simulink model "VirtualADAPT"  (Simulink 8.9)
settings: Solver=ode45  StartTime=0.0  StopTime=inf  RelTol=1e-3  EnableMultiTasking=on
PostLoadFcn:
  | FaultInjectionGUIHandle = FaultInjectionGUI;
InitFcn:
  | VirtualADAPTDataStructure
undefined symbols (not defined in the file or in its folder): ln
libraries: simulink x363
(part 12 of 26)

== system VirtualADAPT/VirtualADAPTv1/PowerDistribution/Inverter2/VoutSetpoint/Fault Inject ==
  Nominal : Inport
  Fault Profile : Inport  Port=2
  Step : Inport  Port=3
  Ramp : Inport  Port=4
  Bias : Inport  Port=5
  stuckAt : Inport  Port=6
  Switch1 : MultiPortSwitch  Inputs=5  SaturateOnIntegerOverflow=on  InputSameDT=on
  Out1 : Outport
  wires:
  Nominal/1 -> Switch1/2
  Fault Profile/1 -> Switch1/1
  Step/1 -> Switch1/3
  Ramp/1 -> Switch1/4
  Bias/1 -> Switch1/5
  stuckAt/1 -> Switch1/6
  Switch1/1 -> Out1/1

== system VirtualADAPT/VirtualADAPTv1/PowerDistribution/Inverter2/Z1/determineState ==
  In1 : Inport
  In2 : Inport  Port=2
  Fcn : Fcn  Expr=(!u(1)&&u(2)) || (u(1)&&(!u(3)))
  Memory : Memory  X0=1
  Mux : Mux  Inputs=3
  Out1 : Outport
  wires:
  Memory/1 -> Mux/1
  In1/1 -> Mux/2
  In2/1 -> Mux/3
  Mux/1 -> Fcn/1
  Fcn/1 -> Memory/1, Out1/1

== system VirtualADAPT/VirtualADAPTv1/PowerDistribution/Inverter2/Z1Delay/C ==
  F : Inport
  Capacitance : Constant  Value=C
  Fault Inject : Subsystem
  Fault Profile : Constant  Value=FP
  InitialCondition : Constant  Value=IC
  Integrator : Integrator  InitialConditionSource=external
  Product1 : Product  SaturateOnIntegerOverflow=on  RndMeth=Zero  InputSameDT=on  OutDataTypeStr=Inherit: Same as first input
  Product2 : Product  SaturateOnIntegerOverflow=on  RndMeth=Zero  InputSameDT=on  OutDataTypeStr=Inherit: Same as first input
  Ramp : simulink/Sources/Ramp  slope=FM  start=FT  X0=C
  Reciprocal : Math  Operator=reciprocal  SaturateOnIntegerOverflow=on
  Step : Step  Time=FT  Before=C  After=(1 + FM)*C  SampleTime=0
  E : Outport
  C : Outport  Port=2
  q : Outport  Port=3
  wires:
  F/1 -> Integrator/1
  InitialCondition/1 -> Product1/1
  Capacitance/1 -> Fault Inject/1(Nominal), Product1/2
  Product1/1 -> Integrator/2
  Integrator/1 -> Product2/1, q/1
  Fault Inject/1(Out1) -> Reciprocal/1, C/1
  Reciprocal/1 -> Product2/2
  Fault Profile/1 -> Fault Inject/2(Fault Profile)
  Step/1 -> Fault Inject/3(Step)
  Ramp/1 -> Fault Inject/4(Ramp)
  Product2/1 -> E/1

== system VirtualADAPT/VirtualADAPTv1/PowerDistribution/Inverter2/Z1Delay/OneJunction ==
  u(1) : Inport
  u(2) : Inport  Port=2
  u(3) : Inport  Port=3
  Adder : Sum  Inputs=-+  SaturateOnIntegerOverflow=on  InputSameDT=on  OutDataTypeStr=Inherit: Same as first input
  Gain : Gain  SaturateOnIntegerOverflow=on  OutDataTypeStr=Inherit: Same as input  ParamDataTypeStr=Inherit: Same as input
  y(1) : Outport
  y(2) : Outport  Port=2
  y(3) : Outport  Port=3
  O : Outport  Port=4
  wires:
  Adder/1 -> Gain/1
  u(2)/1 -> y(1)/1, y(3)/1, O/1
  u(1)/1 -> Adder/1
  Gain/1 -> y(2)/1
  u(3)/1 -> Adder/2

== system VirtualADAPT/VirtualADAPTv1/PowerDistribution/Inverter2/Z1Delay/R ==
  In : Inport
  Bond : Constant  Value=2
  Computing R : Subsystem
  Fault Profile : Constant  Value=FP
  JuncDB : Constant  Value=2
  Junction Type : Constant
  Ramp : simulink/Sources/Ramp  slope=FM  start=FT  X0=R
  Resistor Value : Constant  Value=R
  Step : Step  Time=FT  Before=R  After=(1 + FM)*R  SampleTime=0
  Out : Outport
  R : Outport  Port=2
  wires:
  Computing R/1(Out1) -> Out/1
  In/1 -> Computing R/1(In1)
  Resistor Value/1 -> Computing R/2(RNominal)
  Fault Profile/1 -> Computing R/3(Fault Profile)
  Step/1 -> Computing R/4(Step)
  Ramp/1 -> Computing R/5(Ramp)
  JuncDB/1 -> Computing R/6(JuncDB)
  Bond/1 -> Computing R/7(Bond)
  Junction Type/1 -> Computing R/8(Junction Type)
  Computing R/2(Out2) -> R/1

== system VirtualADAPT/VirtualADAPTv1/PowerDistribution/Inverter2/Z1Delay/Se ==
  F : Inport
  Se : Inport  Port=2
  Bias : Step  Time=FT  After=FM  SampleTime=0
  Fault Inject : Subsystem
  Fault Profile : Constant  Value=FP
  Mult2 : Product  SaturateOnIntegerOverflow=on  RndMeth=Zero  InputSameDT=on  OutDataTypeStr=Inherit: Same as first input
  Multiply : Product  SaturateOnIntegerOverflow=on  RndMeth=Zero  InputSameDT=on  OutDataTypeStr=Inherit: Same as first input
  Ramp : simulink/Sources/Ramp  slope=FM  start=FT  X0=0
  Step : Step  Time=FT  Before=1  After=(1 + FM)  SampleTime=0
  StuckAt : Step  Time=FT  Before=1  After=0  SampleTime=0
  StuckAt2 : Step  Time=FT  After=FM  SampleTime=0
  Sum : Sum  SaturateOnIntegerOverflow=on  InputSameDT=on  OutDataTypeStr=Inherit: Same as first input
  Sum2 : Sum  SaturateOnIntegerOverflow=on  InputSameDT=on  OutDataTypeStr=Inherit: Same as first input
  Sum3 : Sum  SaturateOnIntegerOverflow=on  InputSameDT=on  OutDataTypeStr=Inherit: Same as first input
  E : Outport
  wires:
  Fault Inject/1(Out1) -> E/1
  Se/1 -> Fault Inject/1(Nominal), Multiply/1, Sum/1, Sum2/1, Mult2/1
  Fault Profile/1 -> Fault Inject/2(Fault Profile)
  Step/1 -> Multiply/2
  Ramp/1 -> Sum/2
  Multiply/1 -> Fault Inject/3(Step)
  Sum/1 -> Fault Inject/4(Ramp)
  Bias/1 -> Sum2/2
  Sum2/1 -> Fault Inject/5(Bias)
  StuckAt/1 -> Mult2/2
  Mult2/1 -> Sum3/1
  StuckAt2/1 -> Sum3/2
  Sum3/1 -> Fault Inject/6(stuckAt)

== system VirtualADAPT/VirtualADAPTv1/PowerDistribution/Inverter2/Z1Delay/SignalModFunc ==
  u(1) : Inport
  Function : Fcn  Expr=u(1)
  Mux : Mux  Inputs=1
  y(1) : Outport
  wires:
  u(1)/1 -> Mux/1
  Mux/1 -> Function/1
  Function/1 -> y(1)/1

== system VirtualADAPT/VirtualADAPTv1/PowerDistribution/Inverter2/Z1Delay/ZeroJunction ==
  u(1) : Inport
  u(2) : Inport  Port=2
  Adder : Sum  Inputs=+  SaturateOnIntegerOverflow=on  InputSameDT=on  OutDataTypeStr=Inherit: Same as first input
  Gain : Gain  SaturateOnIntegerOverflow=on  OutDataTypeStr=Inherit: Same as input  ParamDataTypeStr=Inherit: Same as input
  y(1) : Outport
  y(2) : Outport  Port=2
  O : Outport  Port=3
  wires:
  Adder/1 -> Gain/1
  Gain/1 -> y(1)/1
  u(1)/1 -> y(2)/1, O/1
  u(2)/1 -> Adder/1

== system VirtualADAPT/VirtualADAPTv1/PowerDistribution/Inverter2/ZDel/determineState ==
  In1 : Inport
  In2 : Inport  Port=2
  Fcn : Fcn  Expr=(!u(1)&&u(2)) || (u(1)&&(!u(3)))
  Memory : Memory
  Mux : Mux  Inputs=3
  Out1 : Outport
  wires:
  Memory/1 -> Mux/1
  In1/1 -> Mux/2
  In2/1 -> Mux/3
  Mux/1 -> Fcn/1
  Fcn/1 -> Memory/1, Out1/1

== system VirtualADAPT/VirtualADAPTv1/PowerDistribution/Inverter2/ZForR1/determineState ==
  In1 : Inport
  In2 : Inport  Port=2
  Fcn : Fcn  Expr=(!u(1)&&u(2)) || (u(1)&&(!u(3)))
  Memory : Memory  X0=1
  Mux : Mux  Inputs=3
  Out1 : Outport
  wires:
  Memory/1 -> Mux/1
  In1/1 -> Mux/2
  In2/1 -> Mux/3
  Mux/1 -> Fcn/1
  Fcn/1 -> Memory/1, Out1/1

== system VirtualADAPT/VirtualADAPTv1/PowerDistribution/Inverter2/ZForR2/determineState ==
  In1 : Inport
  In2 : Inport  Port=2
  Fcn : Fcn  Expr=(!u(1)&&u(2)) || (u(1)&&(!u(3)))
  Memory : Memory
  Mux : Mux  Inputs=3
  Out1 : Outport
  wires:
  Memory/1 -> Mux/1
  In1/1 -> Mux/2
  In2/1 -> Mux/3
  Mux/1 -> Fcn/1
  Fcn/1 -> Memory/1, Out1/1

== system VirtualADAPT/VirtualADAPTv1/PowerDistribution/Inverter2/ZFreq/determineState ==
  In1 : Inport
  In2 : Inport  Port=2
  Fcn : Fcn  Expr=(!u(1)&&u(2)) || (u(1)&&(!u(3)))
  Memory : Memory
  Mux : Mux  Inputs=3
  Out1 : Outport
  wires:
  Memory/1 -> Mux/1
  In1/1 -> Mux/2
  In2/1 -> Mux/3
  Mux/1 -> Fcn/1
  Fcn/1 -> Memory/1, Out1/1

== system VirtualADAPT/VirtualADAPTv1/PowerDistribution/Inverter2/ZVout/determineState ==
  In1 : Inport
  In2 : Inport  Port=2
  Fcn : Fcn  Expr=(!u(1)&&u(2)) || (u(1)&&(!u(3)))
  Memory : Memory
  Mux : Mux  Inputs=3
  Out1 : Outport
  wires:
  Memory/1 -> Mux/1
  In1/1 -> Mux/2
  In2/1 -> Mux/3
  Mux/1 -> Fcn/1
  Fcn/1 -> Memory/1, Out1/1

== system VirtualADAPT/VirtualADAPTv1/PowerDistribution/SensorE165/MSe/Fault Inject ==
  Nominal : Inport
  Fault Profile : Inport  Port=2
  Step : Inport  Port=3
  Ramp : Inport  Port=4
  Bias : Inport  Port=5
  stuckAt : Inport  Port=6
  Switch1 : MultiPortSwitch  Inputs=5  SaturateOnIntegerOverflow=on  InputSameDT=on
  Out1 : Outport
  wires:
  Nominal/1 -> Switch1/2
  Fault Profile/1 -> Switch1/1
  Step/1 -> Switch1/3
  Ramp/1 -> Switch1/4
  Bias/1 -> Switch1/5
  stuckAt/1 -> Switch1/6
  Switch1/1 -> Out1/1

== system VirtualADAPT/VirtualADAPTv1/PowerDistribution/SensorE165/R/Computing R ==
  In1 : Inport
  RNominal : Inport  Port=2
  Fault Profile : Inport  Port=3
  Step : Inport  Port=4
  Ramp : Inport  Port=5
  JuncDB : Inport  Port=6
  Bond : Inport  Port=7
  Junction Type : Inport  Port=8
  CalcCausality : Fcn  Expr=(u(1) == u(2) && u(3) ==1) || (u(1) !=u(2) && u(3) == 0)
  CalcOutput : Fcn  Expr=(u(1)*u(2)^u(3))
  MinusOne : Constant  Value=-1
  Mux1 : Mux  Inputs=3
  Mux2 : Mux  Inputs=3
  One : Constant
  Switch1 : MultiPortSwitch  SaturateOnIntegerOverflow=on  InputSameDT=on
  Switch2 : MultiPortSwitch  DataPortOrder=Zero-based contiguous  Inputs=2  zeroidx=on  SaturateOnIntegerOverflow=on  InputSameDT=on
  Out1 : Outport
  Out2 : Outport  Port=2
  wires:
  In1/1 -> Mux2/1
  Fault Profile/1 -> Switch1/1
  RNominal/1 -> Switch1/2
  Step/1 -> Switch1/3
  Ramp/1 -> Switch1/4
  Switch1/1 -> Out2/1, Mux2/2
  JuncDB/1 -> Mux1/1
  Bond/1 -> Mux1/2
  Junction Type/1 -> Mux1/3
  Mux1/1 -> CalcCausality/1
  CalcCausality/1 -> Switch2/1
  One/1 -> Switch2/2
  MinusOne/1 -> Switch2/3
  Switch2/1 -> Mux2/3
  Mux2/1 -> CalcOutput/1
  CalcOutput/1 -> Out1/1

== system VirtualADAPT/VirtualADAPTv1/PowerDistribution/SensorE167/MSe/Fault Inject ==
  Nominal : Inport
  Fault Profile : Inport  Port=2
  Step : Inport  Port=3
  Ramp : Inport  Port=4
  Bias : Inport  Port=5
  stuckAt : Inport  Port=6
  Switch1 : MultiPortSwitch  Inputs=5  SaturateOnIntegerOverflow=on  InputSameDT=on
  Out1 : Outport
  wires:
  Nominal/1 -> Switch1/2
  Fault Profile/1 -> Switch1/1
  Step/1 -> Switch1/3
  Ramp/1 -> Switch1/4
  Bias/1 -> Switch1/5
  stuckAt/1 -> Switch1/6
  Switch1/1 -> Out1/1

== system VirtualADAPT/VirtualADAPTv1/PowerDistribution/SensorE167/R/Computing R ==
  In1 : Inport
  RNominal : Inport  Port=2
  Fault Profile : Inport  Port=3
  Step : Inport  Port=4
  Ramp : Inport  Port=5
  JuncDB : Inport  Port=6
  Bond : Inport  Port=7
  Junction Type : Inport  Port=8
  CalcCausality : Fcn  Expr=(u(1) == u(2) && u(3) ==1) || (u(1) !=u(2) && u(3) == 0)
  CalcOutput : Fcn  Expr=(u(1)*u(2)^u(3))
  MinusOne : Constant  Value=-1
  Mux1 : Mux  Inputs=3
  Mux2 : Mux  Inputs=3
  One : Constant
  Switch1 : MultiPortSwitch  SaturateOnIntegerOverflow=on  InputSameDT=on
  Switch2 : MultiPortSwitch  DataPortOrder=Zero-based contiguous  Inputs=2  zeroidx=on  SaturateOnIntegerOverflow=on  InputSameDT=on
  Out1 : Outport
  Out2 : Outport  Port=2
  wires:
  In1/1 -> Mux2/1
  Fault Profile/1 -> Switch1/1
  RNominal/1 -> Switch1/2
  Step/1 -> Switch1/3
  Ramp/1 -> Switch1/4
  Switch1/1 -> Out2/1, Mux2/2
  JuncDB/1 -> Mux1/1
  Bond/1 -> Mux1/2
  Junction Type/1 -> Mux1/3
  Mux1/1 -> CalcCausality/1
  CalcCausality/1 -> Switch2/1
  One/1 -> Switch2/2
  MinusOne/1 -> Switch2/3
  Switch2/1 -> Mux2/3
  Mux2/1 -> CalcOutput/1
  CalcOutput/1 -> Out1/1

== system VirtualADAPT/VirtualADAPTv1/PowerDistribution/SensorE181/MSe/Fault Inject ==
  Nominal : Inport
  Fault Profile : Inport  Port=2
  Step : Inport  Port=3
  Ramp : Inport  Port=4
  Bias : Inport  Port=5
  stuckAt : Inport  Port=6
  Switch1 : MultiPortSwitch  Inputs=5  SaturateOnIntegerOverflow=on  InputSameDT=on
  Out1 : Outport
  wires:
  Nominal/1 -> Switch1/2
  Fault Profile/1 -> Switch1/1
  Step/1 -> Switch1/3
  Ramp/1 -> Switch1/4
  Bias/1 -> Switch1/5
  stuckAt/1 -> Switch1/6
  Switch1/1 -> Out1/1

== system VirtualADAPT/VirtualADAPTv1/PowerDistribution/SensorE181/R/Computing R ==
  In1 : Inport
  RNominal : Inport  Port=2
  Fault Profile : Inport  Port=3
  Step : Inport  Port=4
  Ramp : Inport  Port=5
  JuncDB : Inport  Port=6
  Bond : Inport  Port=7
  Junction Type : Inport  Port=8
  CalcCausality : Fcn  Expr=(u(1) == u(2) && u(3) ==1) || (u(1) !=u(2) && u(3) == 0)
  CalcOutput : Fcn  Expr=(u(1)*u(2)^u(3))
  MinusOne : Constant  Value=-1
  Mux1 : Mux  Inputs=3
  Mux2 : Mux  Inputs=3
  One : Constant
  Switch1 : MultiPortSwitch  SaturateOnIntegerOverflow=on  InputSameDT=on
  Switch2 : MultiPortSwitch  DataPortOrder=Zero-based contiguous  Inputs=2  zeroidx=on  SaturateOnIntegerOverflow=on  InputSameDT=on
  Out1 : Outport
  Out2 : Outport  Port=2
  wires:
  In1/1 -> Mux2/1
  Fault Profile/1 -> Switch1/1
  RNominal/1 -> Switch1/2
  Step/1 -> Switch1/3
  Ramp/1 -> Switch1/4
  Switch1/1 -> Out2/1, Mux2/2
  JuncDB/1 -> Mux1/1
  Bond/1 -> Mux1/2
  Junction Type/1 -> Mux1/3
  Mux1/1 -> CalcCausality/1
  CalcCausality/1 -> Switch2/1
  One/1 -> Switch2/2
  MinusOne/1 -> Switch2/3
  Switch2/1 -> Mux2/3
  Mux2/1 -> CalcOutput/1
  CalcOutput/1 -> Out1/1

== system VirtualADAPT/VirtualADAPTv1/PowerDistribution/SensorE265/MSe/Fault Inject ==
  Nominal : Inport
  Fault Profile : Inport  Port=2
  Step : Inport  Port=3
  Ramp : Inport  Port=4
  Bias : Inport  Port=5
  stuckAt : Inport  Port=6
  Switch1 : MultiPortSwitch  Inputs=5  SaturateOnIntegerOverflow=on  InputSameDT=on
  Out1 : Outport
  wires:
  Nominal/1 -> Switch1/2
  Fault Profile/1 -> Switch1/1
  Step/1 -> Switch1/3
  Ramp/1 -> Switch1/4
  Bias/1 -> Switch1/5
  stuckAt/1 -> Switch1/6
  Switch1/1 -> Out1/1

== system VirtualADAPT/VirtualADAPTv1/PowerDistribution/SensorE265/R/Computing R ==
  In1 : Inport
  RNominal : Inport  Port=2
  Fault Profile : Inport  Port=3
  Step : Inport  Port=4
  Ramp : Inport  Port=5
  JuncDB : Inport  Port=6
  Bond : Inport  Port=7
  Junction Type : Inport  Port=8
  CalcCausality : Fcn  Expr=(u(1) == u(2) && u(3) ==1) || (u(1) !=u(2) && u(3) == 0)
  CalcOutput : Fcn  Expr=(u(1)*u(2)^u(3))
  MinusOne : Constant  Value=-1
  Mux1 : Mux  Inputs=3
  Mux2 : Mux  Inputs=3
  One : Constant
  Switch1 : MultiPortSwitch  SaturateOnIntegerOverflow=on  InputSameDT=on
  Switch2 : MultiPortSwitch  DataPortOrder=Zero-based contiguous  Inputs=2  zeroidx=on  SaturateOnIntegerOverflow=on  InputSameDT=on
  Out1 : Outport
  Out2 : Outport  Port=2
  wires:
  In1/1 -> Mux2/1
  Fault Profile/1 -> Switch1/1
  RNominal/1 -> Switch1/2
  Step/1 -> Switch1/3
  Ramp/1 -> Switch1/4
  Switch1/1 -> Out2/1, Mux2/2
  JuncDB/1 -> Mux1/1
  Bond/1 -> Mux1/2
  Junction Type/1 -> Mux1/3
  Mux1/1 -> CalcCausality/1
  CalcCausality/1 -> Switch2/1
  One/1 -> Switch2/2
  MinusOne/1 -> Switch2/3
  Switch2/1 -> Mux2/3
  Mux2/1 -> CalcOutput/1
  CalcOutput/1 -> Out1/1

== system VirtualADAPT/VirtualADAPTv1/PowerDistribution/SensorE267/MSe/Fault Inject ==
  Nominal : Inport
  Fault Profile : Inport  Port=2
  Step : Inport  Port=3
  Ramp : Inport  Port=4
  Bias : Inport  Port=5
  stuckAt : Inport  Port=6
  Switch1 : MultiPortSwitch  Inputs=5  SaturateOnIntegerOverflow=on  InputSameDT=on
  Out1 : Outport
  wires:
  Nominal/1 -> Switch1/2
  Fault Profile/1 -> Switch1/1
  Step/1 -> Switch1/3
  Ramp/1 -> Switch1/4
  Bias/1 -> Switch1/5
  stuckAt/1 -> Switch1/6
  Switch1/1 -> Out1/1

== system VirtualADAPT/VirtualADAPTv1/PowerDistribution/SensorE267/R/Computing R ==
  In1 : Inport
  RNominal : Inport  Port=2
  Fault Profile : Inport  Port=3
  Step : Inport  Port=4
  Ramp : Inport  Port=5
  JuncDB : Inport  Port=6
  Bond : Inport  Port=7
  Junction Type : Inport  Port=8
  CalcCausality : Fcn  Expr=(u(1) == u(2) && u(3) ==1) || (u(1) !=u(2) && u(3) == 0)
  CalcOutput : Fcn  Expr=(u(1)*u(2)^u(3))
  MinusOne : Constant  Value=-1
  Mux1 : Mux  Inputs=3
  Mux2 : Mux  Inputs=3
  One : Constant
  Switch1 : MultiPortSwitch  SaturateOnIntegerOverflow=on  InputSameDT=on
  Switch2 : MultiPortSwitch  DataPortOrder=Zero-based contiguous  Inputs=2  zeroidx=on  SaturateOnIntegerOverflow=on  InputSameDT=on
  Out1 : Outport
  Out2 : Outport  Port=2
  wires:
  In1/1 -> Mux2/1
  Fault Profile/1 -> Switch1/1
  RNominal/1 -> Switch1/2
  Step/1 -> Switch1/3
  Ramp/1 -> Switch1/4
  Switch1/1 -> Out2/1, Mux2/2
  JuncDB/1 -> Mux1/1
  Bond/1 -> Mux1/2
  Junction Type/1 -> Mux1/3
  Mux1/1 -> CalcCausality/1
  CalcCausality/1 -> Switch2/1
  One/1 -> Switch2/2
  MinusOne/1 -> Switch2/3
  Switch2/1 -> Mux2/3
  Mux2/1 -> CalcOutput/1
  CalcOutput/1 -> Out1/1

== system VirtualADAPT/VirtualADAPTv1/PowerDistribution/SensorE281/MSe/Fault Inject ==
  Nominal : Inport
  Fault Profile : Inport  Port=2
  Step : Inport  Port=3
  Ramp : Inport  Port=4
  Bias : Inport  Port=5
  stuckAt : Inport  Port=6
  Switch1 : MultiPortSwitch  Inputs=5  SaturateOnIntegerOverflow=on  InputSameDT=on
  Out1 : Outport
  wires:
  Nominal/1 -> Switch1/2
  Fault Profile/1 -> Switch1/1
  Step/1 -> Switch1/3
  Ramp/1 -> Switch1/4
  Bias/1 -> Switch1/5
  stuckAt/1 -> Switch1/6
  Switch1/1 -> Out1/1

== system VirtualADAPT/VirtualADAPTv1/PowerDistribution/SensorE281/R/Computing R ==
  In1 : Inport
  RNominal : Inport  Port=2
  Fault Profile : Inport  Port=3
  Step : Inport  Port=4
  Ramp : Inport  Port=5
  JuncDB : Inport  Port=6
  Bond : Inport  Port=7
  Junction Type : Inport  Port=8
  CalcCausality : Fcn  Expr=(u(1) == u(2) && u(3) ==1) || (u(1) !=u(2) && u(3) == 0)
  CalcOutput : Fcn  Expr=(u(1)*u(2)^u(3))
  MinusOne : Constant  Value=-1
  Mux1 : Mux  Inputs=3
  Mux2 : Mux  Inputs=3
  One : Constant
  Switch1 : MultiPortSwitch  SaturateOnIntegerOverflow=on  InputSameDT=on
  Switch2 : MultiPortSwitch  DataPortOrder=Zero-based contiguous  Inputs=2  zeroidx=on  SaturateOnIntegerOverflow=on  InputSameDT=on
  Out1 : Outport
  Out2 : Outport  Port=2
  wires:
  In1/1 -> Mux2/1
  Fault Profile/1 -> Switch1/1
  RNominal/1 -> Switch1/2
  Step/1 -> Switch1/3
  Ramp/1 -> Switch1/4
  Switch1/1 -> Out2/1, Mux2/2
  JuncDB/1 -> Mux1/1
  Bond/1 -> Mux1/2
  Junction Type/1 -> Mux1/3
  Mux1/1 -> CalcCausality/1
  CalcCausality/1 -> Switch2/1
  One/1 -> Switch2/2
  MinusOne/1 -> Switch2/3
  Switch2/1 -> Mux2/3
  Mux2/1 -> CalcOutput/1
  CalcOutput/1 -> Out1/1

== system VirtualADAPT/VirtualADAPTv1/PowerDistribution/SensorISH162/MSe/Fault Inject ==
  Nominal : Inport
  Fault Profile : Inport  Port=2
  Step : Inport  Port=3
  Ramp : Inport  Port=4
  Bias : Inport  Port=5
  stuckAt : Inport  Port=6
  Switch1 : MultiPortSwitch  Inputs=5  SaturateOnIntegerOverflow=on  InputSameDT=on
  Out1 : Outport
  wires:
  Nominal/1 -> Switch1/2
  Fault Profile/1 -> Switch1/1
  Step/1 -> Switch1/3
  Ramp/1 -> Switch1/4
  Bias/1 -> Switch1/5
  stuckAt/1 -> Switch1/6
  Switch1/1 -> Out1/1

== system VirtualADAPT/VirtualADAPTv1/PowerDistribution/SensorISH162/R/Computing R ==
  In1 : Inport
  RNominal : Inport  Port=2
  Fault Profile : Inport  Port=3
  Step : Inport  Port=4
  Ramp : Inport  Port=5
  JuncDB : Inport  Port=6
  Bond : Inport  Port=7
  Junction Type : Inport  Port=8
  CalcCausality : Fcn  Expr=(u(1) == u(2) && u(3) ==1) || (u(1) !=u(2) && u(3) == 0)
  CalcOutput : Fcn  Expr=(u(1)*u(2)^u(3))
  MinusOne : Constant  Value=-1
  Mux1 : Mux  Inputs=3
  Mux2 : Mux  Inputs=3
  One : Constant
  Switch1 : MultiPortSwitch  SaturateOnIntegerOverflow=on  InputSameDT=on
  Switch2 : MultiPortSwitch  DataPortOrder=Zero-based contiguous  Inputs=2  zeroidx=on  SaturateOnIntegerOverflow=on  InputSameDT=on
  Out1 : Outport
  Out2 : Outport  Port=2
  wires:
  In1/1 -> Mux2/1
  Fault Profile/1 -> Switch1/1
  RNominal/1 -> Switch1/2
  Step/1 -> Switch1/3
  Ramp/1 -> Switch1/4
  Switch1/1 -> Out2/1, Mux2/2
  JuncDB/1 -> Mux1/1
  Bond/1 -> Mux1/2
  Junction Type/1 -> Mux1/3
  Mux1/1 -> CalcCausality/1
  CalcCausality/1 -> Switch2/1
  One/1 -> Switch2/2
  MinusOne/1 -> Switch2/3
  Switch2/1 -> Mux2/3
  Mux2/1 -> CalcOutput/1
  CalcOutput/1 -> Out1/1

== system VirtualADAPT/VirtualADAPTv1/PowerDistribution/SensorISH166/MSe/Fault Inject ==
  Nominal : Inport
  Fault Profile : Inport  Port=2
  Step : Inport  Port=3
  Ramp : Inport  Port=4
  Bias : Inport  Port=5
  stuckAt : Inport  Port=6
  Switch1 : MultiPortSwitch  Inputs=5  SaturateOnIntegerOverflow=on  InputSameDT=on
  Out1 : Outport
  wires:
  Nominal/1 -> Switch1/2
  Fault Profile/1 -> Switch1/1
  Step/1 -> Switch1/3
  Ramp/1 -> Switch1/4
  Bias/1 -> Switch1/5
  stuckAt/1 -> Switch1/6
  Switch1/1 -> Out1/1

== system VirtualADAPT/VirtualADAPTv1/PowerDistribution/SensorISH166/R/Computing R ==
  In1 : Inport
  RNominal : Inport  Port=2
  Fault Profile : Inport  Port=3
  Step : Inport  Port=4
  Ramp : Inport  Port=5
  JuncDB : Inport  Port=6
  Bond : Inport  Port=7
  Junction Type : Inport  Port=8
  CalcCausality : Fcn  Expr=(u(1) == u(2) && u(3) ==1) || (u(1) !=u(2) && u(3) == 0)
  CalcOutput : Fcn  Expr=(u(1)*u(2)^u(3))
  MinusOne : Constant  Value=-1
  Mux1 : Mux  Inputs=3
  Mux2 : Mux  Inputs=3
  One : Constant
  Switch1 : MultiPortSwitch  SaturateOnIntegerOverflow=on  InputSameDT=on
  Switch2 : MultiPortSwitch  DataPortOrder=Zero-based contiguous  Inputs=2  zeroidx=on  SaturateOnIntegerOverflow=on  InputSameDT=on
  Out1 : Outport
  Out2 : Outport  Port=2
  wires:
  In1/1 -> Mux2/1
  Fault Profile/1 -> Switch1/1
  RNominal/1 -> Switch1/2
  Step/1 -> Switch1/3
  Ramp/1 -> Switch1/4
  Switch1/1 -> Out2/1, Mux2/2
  JuncDB/1 -> Mux1/1
  Bond/1 -> Mux1/2
  Junction Type/1 -> Mux1/3
  Mux1/1 -> CalcCausality/1
  CalcCausality/1 -> Switch2/1
  One/1 -> Switch2/2
  MinusOne/1 -> Switch2/3
  Switch2/1 -> Mux2/3
  Mux2/1 -> CalcOutput/1
  CalcOutput/1 -> Out1/1

== system VirtualADAPT/VirtualADAPTv1/PowerDistribution/SensorISH180/MSe/Fault Inject ==
  Nominal : Inport
  Fault Profile : Inport  Port=2
  Step : Inport  Port=3
  Ramp : Inport  Port=4
  Bias : Inport  Port=5
  stuckAt : Inport  Port=6
  Switch1 : MultiPortSwitch  Inputs=5  SaturateOnIntegerOverflow=on  InputSameDT=on
  Out1 : Outport
  wires:
  Nominal/1 -> Switch1/2
  Fault Profile/1 -> Switch1/1
  Step/1 -> Switch1/3
  Ramp/1 -> Switch1/4
  Bias/1 -> Switch1/5
  stuckAt/1 -> Switch1/6
  Switch1/1 -> Out1/1

== system VirtualADAPT/VirtualADAPTv1/PowerDistribution/SensorISH180/R/Computing R ==
  In1 : Inport
  RNominal : Inport  Port=2
  Fault Profile : Inport  Port=3
  Step : Inport  Port=4
  Ramp : Inport  Port=5
  JuncDB : Inport  Port=6
  Bond : Inport  Port=7
  Junction Type : Inport  Port=8
  CalcCausality : Fcn  Expr=(u(1) == u(2) && u(3) ==1) || (u(1) !=u(2) && u(3) == 0)
  CalcOutput : Fcn  Expr=(u(1)*u(2)^u(3))
  MinusOne : Constant  Value=-1
  Mux1 : Mux  Inputs=3
  Mux2 : Mux  Inputs=3
  One : Constant
  Switch1 : MultiPortSwitch  SaturateOnIntegerOverflow=on  InputSameDT=on
  Switch2 : MultiPortSwitch  DataPortOrder=Zero-based contiguous  Inputs=2  zeroidx=on  SaturateOnIntegerOverflow=on  InputSameDT=on
  Out1 : Outport
  Out2 : Outport  Port=2
  wires:
  In1/1 -> Mux2/1
  Fault Profile/1 -> Switch1/1
  RNominal/1 -> Switch1/2
  Step/1 -> Switch1/3
  Ramp/1 -> Switch1/4
  Switch1/1 -> Out2/1, Mux2/2
  JuncDB/1 -> Mux1/1
  Bond/1 -> Mux1/2
  Junction Type/1 -> Mux1/3
  Mux1/1 -> CalcCausality/1
  CalcCausality/1 -> Switch2/1
  One/1 -> Switch2/2
  MinusOne/1 -> Switch2/3
  Switch2/1 -> Mux2/3
  Mux2/1 -> CalcOutput/1
  CalcOutput/1 -> Out1/1

== system VirtualADAPT/VirtualADAPTv1/PowerDistribution/SensorISH262/MSe/Fault Inject ==
  Nominal : Inport
  Fault Profile : Inport  Port=2
  Step : Inport  Port=3
  Ramp : Inport  Port=4
  Bias : Inport  Port=5
  stuckAt : Inport  Port=6
  Switch1 : MultiPortSwitch  Inputs=5  SaturateOnIntegerOverflow=on  InputSameDT=on
  Out1 : Outport
  wires:
  Nominal/1 -> Switch1/2
  Fault Profile/1 -> Switch1/1
  Step/1 -> Switch1/3
  Ramp/1 -> Switch1/4
  Bias/1 -> Switch1/5
  stuckAt/1 -> Switch1/6
  Switch1/1 -> Out1/1

== system VirtualADAPT/VirtualADAPTv1/PowerDistribution/SensorISH262/R/Computing R ==
  In1 : Inport
  RNominal : Inport  Port=2
  Fault Profile : Inport  Port=3
  Step : Inport  Port=4
  Ramp : Inport  Port=5
  JuncDB : Inport  Port=6
  Bond : Inport  Port=7
  Junction Type : Inport  Port=8
  CalcCausality : Fcn  Expr=(u(1) == u(2) && u(3) ==1) || (u(1) !=u(2) && u(3) == 0)
  CalcOutput : Fcn  Expr=(u(1)*u(2)^u(3))
  MinusOne : Constant  Value=-1
  Mux1 : Mux  Inputs=3
  Mux2 : Mux  Inputs=3
  One : Constant
  Switch1 : MultiPortSwitch  SaturateOnIntegerOverflow=on  InputSameDT=on
  Switch2 : MultiPortSwitch  DataPortOrder=Zero-based contiguous  Inputs=2  zeroidx=on  SaturateOnIntegerOverflow=on  InputSameDT=on
  Out1 : Outport
  Out2 : Outport  Port=2
  wires:
  In1/1 -> Mux2/1
  Fault Profile/1 -> Switch1/1
  RNominal/1 -> Switch1/2
  Step/1 -> Switch1/3
  Ramp/1 -> Switch1/4
  Switch1/1 -> Out2/1, Mux2/2
  JuncDB/1 -> Mux1/1
  Bond/1 -> Mux1/2
  Junction Type/1 -> Mux1/3
  Mux1/1 -> CalcCausality/1
  CalcCausality/1 -> Switch2/1
  One/1 -> Switch2/2
  MinusOne/1 -> Switch2/3
  Switch2/1 -> Mux2/3
  Mux2/1 -> CalcOutput/1
  CalcOutput/1 -> Out1/1

== system VirtualADAPT/VirtualADAPTv1/PowerDistribution/SensorISH266/MSe/Fault Inject ==
  Nominal : Inport
  Fault Profile : Inport  Port=2
  Step : Inport  Port=3
  Ramp : Inport  Port=4
  Bias : Inport  Port=5
  stuckAt : Inport  Port=6
  Switch1 : MultiPortSwitch  Inputs=5  SaturateOnIntegerOverflow=on  InputSameDT=on
  Out1 : Outport
  wires:
  Nominal/1 -> Switch1/2
  Fault Profile/1 -> Switch1/1
  Step/1 -> Switch1/3
  Ramp/1 -> Switch1/4
  Bias/1 -> Switch1/5
  stuckAt/1 -> Switch1/6
  Switch1/1 -> Out1/1

== system VirtualADAPT/VirtualADAPTv1/PowerDistribution/SensorISH266/R/Computing R ==
  In1 : Inport
  RNominal : Inport  Port=2
  Fault Profile : Inport  Port=3
  Step : Inport  Port=4
  Ramp : Inport  Port=5
  JuncDB : Inport  Port=6
  Bond : Inport  Port=7
  Junction Type : Inport  Port=8
  CalcCausality : Fcn  Expr=(u(1) == u(2) && u(3) ==1) || (u(1) !=u(2) && u(3) == 0)
  CalcOutput : Fcn  Expr=(u(1)*u(2)^u(3))
  MinusOne : Constant  Value=-1
  Mux1 : Mux  Inputs=3
  Mux2 : Mux  Inputs=3
  One : Constant
  Switch1 : MultiPortSwitch  SaturateOnIntegerOverflow=on  InputSameDT=on
  Switch2 : MultiPortSwitch  DataPortOrder=Zero-based contiguous  Inputs=2  zeroidx=on  SaturateOnIntegerOverflow=on  InputSameDT=on
  Out1 : Outport
  Out2 : Outport  Port=2
  wires:
  In1/1 -> Mux2/1
  Fault Profile/1 -> Switch1/1
  RNominal/1 -> Switch1/2
  Step/1 -> Switch1/3
  Ramp/1 -> Switch1/4
  Switch1/1 -> Out2/1, Mux2/2
  JuncDB/1 -> Mux1/1
  Bond/1 -> Mux1/2
  Junction Type/1 -> Mux1/3
  Mux1/1 -> CalcCausality/1
  CalcCausality/1 -> Switch2/1
  One/1 -> Switch2/2
  MinusOne/1 -> Switch2/3
  Switch2/1 -> Mux2/3
  Mux2/1 -> CalcOutput/1
  CalcOutput/1 -> Out1/1

== system VirtualADAPT/VirtualADAPTv1/PowerDistribution/SensorISH280/MSe/Fault Inject ==
  Nominal : Inport
  Fault Profile : Inport  Port=2
  Step : Inport  Port=3
  Ramp : Inport  Port=4
  Bias : Inport  Port=5
  stuckAt : Inport  Port=6
  Switch1 : MultiPortSwitch  Inputs=5  SaturateOnIntegerOverflow=on  InputSameDT=on
  Out1 : Outport
  wires:
  Nominal/1 -> Switch1/2
  Fault Profile/1 -> Switch1/1
  Step/1 -> Switch1/3
  Ramp/1 -> Switch1/4
  Bias/1 -> Switch1/5
  stuckAt/1 -> Switch1/6
  Switch1/1 -> Out1/1

== system VirtualADAPT/VirtualADAPTv1/PowerDistribution/SensorISH280/R/Computing R ==
  In1 : Inport
  RNominal : Inport  Port=2
  Fault Profile : Inport  Port=3
  Step : Inport  Port=4
  Ramp : Inport  Port=5
  JuncDB : Inport  Port=6
  Bond : Inport  Port=7
  Junction Type : Inport  Port=8
  CalcCausality : Fcn  Expr=(u(1) == u(2) && u(3) ==1) || (u(1) !=u(2) && u(3) == 0)
  CalcOutput : Fcn  Expr=(u(1)*u(2)^u(3))
  MinusOne : Constant  Value=-1
  Mux1 : Mux  Inputs=3
  Mux2 : Mux  Inputs=3
  One : Constant
  Switch1 : MultiPortSwitch  SaturateOnIntegerOverflow=on  InputSameDT=on
  Switch2 : MultiPortSwitch  DataPortOrder=Zero-based contiguous  Inputs=2  zeroidx=on  SaturateOnIntegerOverflow=on  InputSameDT=on
  Out1 : Outport
  Out2 : Outport  Port=2
  wires:
  In1/1 -> Mux2/1
  Fault Profile/1 -> Switch1/1
  RNominal/1 -> Switch1/2
  Step/1 -> Switch1/3
  Ramp/1 -> Switch1/4
  Switch1/1 -> Out2/1, Mux2/2
  JuncDB/1 -> Mux1/1
  Bond/1 -> Mux1/2
  Junction Type/1 -> Mux1/3
  Mux1/1 -> CalcCausality/1
  CalcCausality/1 -> Switch2/1
  One/1 -> Switch2/2
  MinusOne/1 -> Switch2/3
  Switch2/1 -> Mux2/3
  Mux2/1 -> CalcOutput/1
  CalcOutput/1 -> Out1/1

== system VirtualADAPT/VirtualADAPTv1/PowerDistribution/SensorIT167/MSe/Fault Inject ==
  Nominal : Inport
  Fault Profile : Inport  Port=2
  Step : Inport  Port=3
  Ramp : Inport  Port=4
  Bias : Inport  Port=5
  stuckAt : Inport  Port=6
  Switch1 : MultiPortSwitch  Inputs=5  SaturateOnIntegerOverflow=on  InputSameDT=on
  Out1 : Outport
  wires:
  Nominal/1 -> Switch1/2
  Fault Profile/1 -> Switch1/1
  Step/1 -> Switch1/3
  Ramp/1 -> Switch1/4
  Bias/1 -> Switch1/5
  stuckAt/1 -> Switch1/6
  Switch1/1 -> Out1/1

== system VirtualADAPT/VirtualADAPTv1/PowerDistribution/SensorIT167/R/Computing R ==
  In1 : Inport
  RNominal : Inport  Port=2
  Fault Profile : Inport  Port=3
  Step : Inport  Port=4
  Ramp : Inport  Port=5
  JuncDB : Inport  Port=6
  Bond : Inport  Port=7
  Junction Type : Inport  Port=8
  CalcCausality : Fcn  Expr=(u(1) == u(2) && u(3) ==1) || (u(1) !=u(2) && u(3) == 0)
  CalcOutput : Fcn  Expr=(u(1)*u(2)^u(3))
  MinusOne : Constant  Value=-1
  Mux1 : Mux  Inputs=3
  Mux2 : Mux  Inputs=3
  One : Constant
  Switch1 : MultiPortSwitch  SaturateOnIntegerOverflow=on  InputSameDT=on
  Switch2 : MultiPortSwitch  DataPortOrder=Zero-based contiguous  Inputs=2  zeroidx=on  SaturateOnIntegerOverflow=on  InputSameDT=on
  Out1 : Outport
  Out2 : Outport  Port=2
  wires:
  In1/1 -> Mux2/1
  Fault Profile/1 -> Switch1/1
  RNominal/1 -> Switch1/2
  Step/1 -> Switch1/3
  Ramp/1 -> Switch1/4
  Switch1/1 -> Out2/1, Mux2/2
  JuncDB/1 -> Mux1/1
  Bond/1 -> Mux1/2
  Junction Type/1 -> Mux1/3
  Mux1/1 -> CalcCausality/1
  CalcCausality/1 -> Switch2/1
  One/1 -> Switch2/2
  MinusOne/1 -> Switch2/3
  Switch2/1 -> Mux2/3
  Mux2/1 -> CalcOutput/1
  CalcOutput/1 -> Out1/1

== system VirtualADAPT/VirtualADAPTv1/PowerDistribution/SensorIT181/MSe/Fault Inject ==
  Nominal : Inport
  Fault Profile : Inport  Port=2
  Step : Inport  Port=3
  Ramp : Inport  Port=4
  Bias : Inport  Port=5
  stuckAt : Inport  Port=6
  Switch1 : MultiPortSwitch  Inputs=5  SaturateOnIntegerOverflow=on  InputSameDT=on
  Out1 : Outport
  wires:
  Nominal/1 -> Switch1/2
  Fault Profile/1 -> Switch1/1
  Step/1 -> Switch1/3
  Ramp/1 -> Switch1/4
  Bias/1 -> Switch1/5
  stuckAt/1 -> Switch1/6
  Switch1/1 -> Out1/1

== system VirtualADAPT/VirtualADAPTv1/PowerDistribution/SensorIT181/R/Computing R ==
  In1 : Inport
  RNominal : Inport  Port=2
  Fault Profile : Inport  Port=3
  Step : Inport  Port=4
  Ramp : Inport  Port=5
  JuncDB : Inport  Port=6
  Bond : Inport  Port=7
  Junction Type : Inport  Port=8
  CalcCausality : Fcn  Expr=(u(1) == u(2) && u(3) ==1) || (u(1) !=u(2) && u(3) == 0)
  CalcOutput : Fcn  Expr=(u(1)*u(2)^u(3))
  MinusOne : Constant  Value=-1
  Mux1 : Mux  Inputs=3
  Mux2 : Mux  Inputs=3
  One : Constant
  Switch1 : MultiPortSwitch  SaturateOnIntegerOverflow=on  InputSameDT=on
  Switch2 : MultiPortSwitch  DataPortOrder=Zero-based contiguous  Inputs=2  zeroidx=on  SaturateOnIntegerOverflow=on  InputSameDT=on
  Out1 : Outport
  Out2 : Outport  Port=2
  wires:
  In1/1 -> Mux2/1
  Fault Profile/1 -> Switch1/1
  RNominal/1 -> Switch1/2
  Step/1 -> Switch1/3
  Ramp/1 -> Switch1/4
  Switch1/1 -> Out2/1, Mux2/2
  JuncDB/1 -> Mux1/1
  Bond/1 -> Mux1/2
  Junction Type/1 -> Mux1/3
  Mux1/1 -> CalcCausality/1
  CalcCausality/1 -> Switch2/1
  One/1 -> Switch2/2
  MinusOne/1 -> Switch2/3
  Switch2/1 -> Mux2/3
  Mux2/1 -> CalcOutput/1
  CalcOutput/1 -> Out1/1

== system VirtualADAPT/VirtualADAPTv1/PowerDistribution/SensorIT267/MSe/Fault Inject ==
  Nominal : Inport
  Fault Profile : Inport  Port=2
  Step : Inport  Port=3
  Ramp : Inport  Port=4
  Bias : Inport  Port=5
  stuckAt : Inport  Port=6
  Switch1 : MultiPortSwitch  Inputs=5  SaturateOnIntegerOverflow=on  InputSameDT=on
  Out1 : Outport
  wires:
  Nominal/1 -> Switch1/2
  Fault Profile/1 -> Switch1/1
  Step/1 -> Switch1/3
  Ramp/1 -> Switch1/4
  Bias/1 -> Switch1/5
  stuckAt/1 -> Switch1/6
  Switch1/1 -> Out1/1

== system VirtualADAPT/VirtualADAPTv1/PowerDistribution/SensorIT267/R/Computing R ==
  In1 : Inport
  RNominal : Inport  Port=2
  Fault Profile : Inport  Port=3
  Step : Inport  Port=4
  Ramp : Inport  Port=5
  JuncDB : Inport  Port=6
  Bond : Inport  Port=7
  Junction Type : Inport  Port=8
  CalcCausality : Fcn  Expr=(u(1) == u(2) && u(3) ==1) || (u(1) !=u(2) && u(3) == 0)
  CalcOutput : Fcn  Expr=(u(1)*u(2)^u(3))
  MinusOne : Constant  Value=-1
  Mux1 : Mux  Inputs=3
  Mux2 : Mux  Inputs=3
  One : Constant
  Switch1 : MultiPortSwitch  SaturateOnIntegerOverflow=on  InputSameDT=on
  Switch2 : MultiPortSwitch  DataPortOrder=Zero-based contiguous  Inputs=2  zeroidx=on  SaturateOnIntegerOverflow=on  InputSameDT=on
  Out1 : Outport
  Out2 : Outport  Port=2
  wires:
  In1/1 -> Mux2/1
  Fault Profile/1 -> Switch1/1
  RNominal/1 -> Switch1/2
  Step/1 -> Switch1/3
  Ramp/1 -> Switch1/4
  Switch1/1 -> Out2/1, Mux2/2
  JuncDB/1 -> Mux1/1
  Bond/1 -> Mux1/2
  Junction Type/1 -> Mux1/3
  Mux1/1 -> CalcCausality/1
  CalcCausality/1 -> Switch2/1
  One/1 -> Switch2/2
  MinusOne/1 -> Switch2/3
  Switch2/1 -> Mux2/3
  Mux2/1 -> CalcOutput/1
  CalcOutput/1 -> Out1/1

== system VirtualADAPT/VirtualADAPTv1/PowerDistribution/SensorIT281/MSe/Fault Inject ==
  Nominal : Inport
  Fault Profile : Inport  Port=2
  Step : Inport  Port=3
  Ramp : Inport  Port=4
  Bias : Inport  Port=5
  stuckAt : Inport  Port=6
  Switch1 : MultiPortSwitch  Inputs=5  SaturateOnIntegerOverflow=on  InputSameDT=on
  Out1 : Outport
  wires:
  Nominal/1 -> Switch1/2
  Fault Profile/1 -> Switch1/1
  Step/1 -> Switch1/3
  Ramp/1 -> Switch1/4
  Bias/1 -> Switch1/5
  stuckAt/1 -> Switch1/6
  Switch1/1 -> Out1/1

== system VirtualADAPT/VirtualADAPTv1/PowerDistribution/SensorIT281/R/Computing R ==
  In1 : Inport
  RNominal : Inport  Port=2
  Fault Profile : Inport  Port=3
  Step : Inport  Port=4
  Ramp : Inport  Port=5
  JuncDB : Inport  Port=6
  Bond : Inport  Port=7
  Junction Type : Inport  Port=8
  CalcCausality : Fcn  Expr=(u(1) == u(2) && u(3) ==1) || (u(1) !=u(2) && u(3) == 0)
  CalcOutput : Fcn  Expr=(u(1)*u(2)^u(3))
  MinusOne : Constant  Value=-1
  Mux1 : Mux  Inputs=3
  Mux2 : Mux  Inputs=3
  One : Constant
  Switch1 : MultiPortSwitch  SaturateOnIntegerOverflow=on  InputSameDT=on
  Switch2 : MultiPortSwitch  DataPortOrder=Zero-based contiguous  Inputs=2  zeroidx=on  SaturateOnIntegerOverflow=on  InputSameDT=on
  Out1 : Outport
  Out2 : Outport  Port=2
  wires:
  In1/1 -> Mux2/1
  Fault Profile/1 -> Switch1/1
  RNominal/1 -> Switch1/2
  Step/1 -> Switch1/3
  Ramp/1 -> Switch1/4
  Switch1/1 -> Out2/1, Mux2/2
  JuncDB/1 -> Mux1/1
  Bond/1 -> Mux1/2
  Junction Type/1 -> Mux1/3
  Mux1/1 -> CalcCausality/1
  CalcCausality/1 -> Switch2/1
  One/1 -> Switch2/2
  MinusOne/1 -> Switch2/3
  Switch2/1 -> Mux2/3
  Mux2/1 -> CalcOutput/1
  CalcOutput/1 -> Out1/1

== system VirtualADAPT/VirtualADAPTv1/PowerDistribution/SensorST165/MSe/Fault Inject ==
  Nominal : Inport
  Fault Profile : Inport  Port=2
  Step : Inport  Port=3
  Ramp : Inport  Port=4
  Bias : Inport  Port=5
  stuckAt : Inport  Port=6
  Switch1 : MultiPortSwitch  Inputs=5  SaturateOnIntegerOverflow=on  InputSameDT=on
  Out1 : Outport
  wires:
  Nominal/1 -> Switch1/2
  Fault Profile/1 -> Switch1/1
  Step/1 -> Switch1/3
  Ramp/1 -> Switch1/4
  Bias/1 -> Switch1/5
  stuckAt/1 -> Switch1/6
  Switch1/1 -> Out1/1

== system VirtualADAPT/VirtualADAPTv1/PowerDistribution/SensorST165/R/Computing R ==
  In1 : Inport
  RNominal : Inport  Port=2
  Fault Profile : Inport  Port=3
  Step : Inport  Port=4
  Ramp : Inport  Port=5
  JuncDB : Inport  Port=6
  Bond : Inport  Port=7
  Junction Type : Inport  Port=8
  CalcCausality : Fcn  Expr=(u(1) == u(2) && u(3) ==1) || (u(1) !=u(2) && u(3) == 0)
  CalcOutput : Fcn  Expr=(u(1)*u(2)^u(3))
  MinusOne : Constant  Value=-1
  Mux1 : Mux  Inputs=3
  Mux2 : Mux  Inputs=3
  One : Constant
  Switch1 : MultiPortSwitch  SaturateOnIntegerOverflow=on  InputSameDT=on
  Switch2 : MultiPortSwitch  DataPortOrder=Zero-based contiguous  Inputs=2  zeroidx=on  SaturateOnIntegerOverflow=on  InputSameDT=on
  Out1 : Outport
  Out2 : Outport  Port=2
  wires:
  In1/1 -> Mux2/1
  Fault Profile/1 -> Switch1/1
  RNominal/1 -> Switch1/2
  Step/1 -> Switch1/3
  Ramp/1 -> Switch1/4
  Switch1/1 -> Out2/1, Mux2/2
  JuncDB/1 -> Mux1/1
  Bond/1 -> Mux1/2
  Junction Type/1 -> Mux1/3
  Mux1/1 -> CalcCausality/1
  CalcCausality/1 -> Switch2/1
  One/1 -> Switch2/2
  MinusOne/1 -> Switch2/3
  Switch2/1 -> Mux2/3
  Mux2/1 -> CalcOutput/1
  CalcOutput/1 -> Out1/1

== system VirtualADAPT/VirtualADAPTv1/PowerDistribution/SensorST265/MSe/Fault Inject ==
  Nominal : Inport
  Fault Profile : Inport  Port=2
  Step : Inport  Port=3
  Ramp : Inport  Port=4
  Bias : Inport  Port=5
  stuckAt : Inport  Port=6
  Switch1 : MultiPortSwitch  Inputs=5  SaturateOnIntegerOverflow=on  InputSameDT=on
  Out1 : Outport
  wires:
  Nominal/1 -> Switch1/2
  Fault Profile/1 -> Switch1/1
  Step/1 -> Switch1/3
  Ramp/1 -> Switch1/4
  Bias/1 -> Switch1/5
  stuckAt/1 -> Switch1/6
  Switch1/1 -> Out1/1

== system VirtualADAPT/VirtualADAPTv1/PowerDistribution/SensorST265/R/Computing R ==
  In1 : Inport
  RNominal : Inport  Port=2
  Fault Profile : Inport  Port=3
  Step : Inport  Port=4
  Ramp : Inport  Port=5
  JuncDB : Inport  Port=6
  Bond : Inport  Port=7
  Junction Type : Inport  Port=8
  CalcCausality : Fcn  Expr=(u(1) == u(2) && u(3) ==1) || (u(1) !=u(2) && u(3) == 0)
  CalcOutput : Fcn  Expr=(u(1)*u(2)^u(3))
  MinusOne : Constant  Value=-1
  Mux1 : Mux  Inputs=3
  Mux2 : Mux  Inputs=3
  One : Constant
  Switch1 : MultiPortSwitch  SaturateOnIntegerOverflow=on  InputSameDT=on
  Switch2 : MultiPortSwitch  DataPortOrder=Zero-based contiguous  Inputs=2  zeroidx=on  SaturateOnIntegerOverflow=on  InputSameDT=on
  Out1 : Outport
  Out2 : Outport  Port=2
  wires:
  In1/1 -> Mux2/1
  Fault Profile/1 -> Switch1/1
  RNominal/1 -> Switch1/2
  Step/1 -> Switch1/3
  Ramp/1 -> Switch1/4
  Switch1/1 -> Out2/1, Mux2/2
  JuncDB/1 -> Mux1/1
  Bond/1 -> Mux1/2
  Junction Type/1 -> Mux1/3
  Mux1/1 -> CalcCausality/1
  CalcCausality/1 -> Switch2/1
  One/1 -> Switch2/2
  MinusOne/1 -> Switch2/3
  Switch2/1 -> Mux2/3
  Mux2/1 -> CalcOutput/1
  CalcOutput/1 -> Out1/1

== system VirtualADAPT/VirtualADAPTv1/PowerStorage/Battery1Subsystem/Battery1/Charge ==
  Temp : Inport
  Ibatt : Inport  Port=2
  q : Inport  Port=3
  C : Subsystem  mask{C=1, IC=0, FP=Nominal, FT=0, FM=0}
      mask prompts: C "Value of C:"; IC "Initial Condition:"; FP "Fault Profile:"; FT "Fault Injection Time:"; FM "Fault Magnitude/Slope"
      mask TypeOptions FP: Nominal | Abrupt | Incipient
      mask Display: image(imread('C.png','BackgroundColor',[1 1 1]))
  ComputeDOC : Subsystem
      mask Display: image(imread('fx.png','BackgroundColor',[1 1 1]))
  ComputeSOC : Subsystem
      mask Display: image(imread('fx.png','BackgroundColor',[1 1 1]))
  Function : Subsystem
      mask Display: image(imread('fx.png','BackgroundColor',[1 1 1]))
  IbattNegative : Subsystem
      mask Display: image(imread('fx.png','BackgroundColor',[1 1 1]))
  Imod : Subsystem
      mask Display: image(imread('1.png','BackgroundColor',[1 1 1]))
  Sf : Subsystem  mask{FP=Nominal, FT=0, FM=0}
      mask prompts: FP "Fault Profile:"; FT "Fault Injection Time:"; FM "Fault Magnitude/Slope/Bias/StuckAtValue"
      mask TypeOptions FP: Nominal | Abrupt | Incipient | Bias | StuckAt
      mask Display: image(imread('MSf.png','BackgroundColor',[1 1 1]))
  ZeroJunction : Subsystem
      mask Display: image(imread('0.png','BackgroundColor',[1 1 1]))
  SOC : Outport
  DOC : Outport  Port=2
  wires:
  ZeroJunction/1(y(1)) -> C/1(F)
  C/1(E) -> ZeroJunction/1(u(1))
  Imod/1(y(1)) -> ZeroJunction/2(u(2))
  ZeroJunction/2(y(2)) -> Imod/1(u(1))
  Sf/1(F) -> Imod/2(u(2))
  Imod/2(y(2)) -> Sf/1(E)
  Function/1(y(1)) -> Sf/2(Sf)
  IbattNegative/1(y(1)) -> Imod/3(u(3))
  Temp/1 -> ComputeSOC/1(u(1)), ComputeDOC/2(u(2))
  Ibatt/1 -> IbattNegative/1(u(1)), Function/1(u(1))
  Imod/3(O) -> ComputeDOC/3(u(3))
  ComputeDOC/1(y(1)) -> DOC/1
  ComputeSOC/1(y(1)) -> SOC/1
  q/1 -> ComputeSOC/2(u(2)), ComputeDOC/1(u(1))

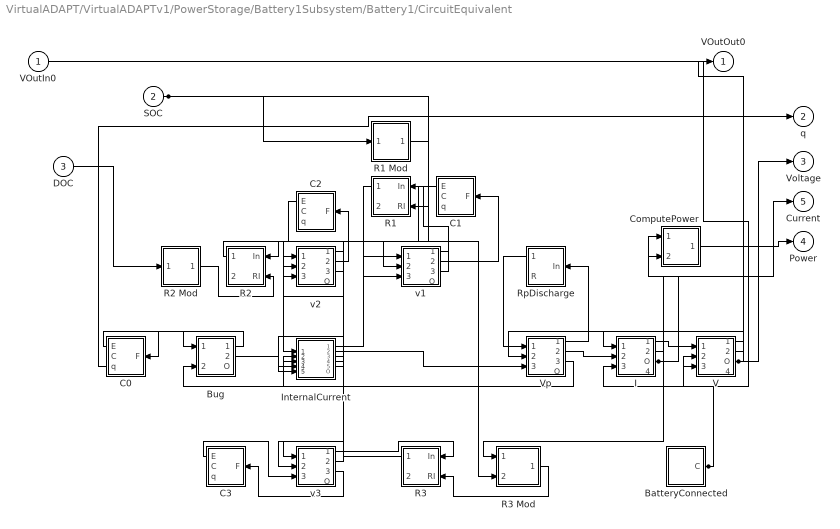
== system VirtualADAPT/VirtualADAPTv1/PowerStorage/Battery1Subsystem/Battery1/CircuitEquivalent ==
  VOutIn0 : Inport
  SOC : Inport  Port=2
  DOC : Inport  Port=3
  BatteryConnected : Subsystem  mask{Constant=1, FP=Nominal, FT=0, FM=0}
      mask prompts: Constant "Value of Constant:"; FP "Fault Profile:"; FT "Fault Injection Time:"; FM "Fault Magnitude/Slope"
      mask TypeOptions FP: Nominal | Abrupt | Incipient
      mask Display: image(imread('Constant.png','BackgroundColor',[1 1 1]))
  Bug : Subsystem
      mask Display: image(imread('0.png','BackgroundColor',[1 1 1]))
  C0 : Subsystem  mask{C=106361, IC=24.2, FP=Nominal, FT=0, FM=99}
      mask prompts: C "Value of C:"; IC "Initial Condition:"; FP "Fault Profile:"; FT "Fault Injection Time:"; FM "Fault Magnitude/Slope"
      mask TypeOptions FP: Nominal | Abrupt | Incipient
      mask Display: image(imread('C.png','BackgroundColor',[1 1 1]))
  C1 : Subsystem  mask{C=51.079, IC=0, FP=Nominal, FT=0, FM=0}
      mask prompts: C "Value of C:"; IC "Initial Condition:"; FP "Fault Profile:"; FT "Fault Injection Time:"; FM "Fault Magnitude/Slope"
      mask TypeOptions FP: Nominal | Abrupt | Incipient
      mask Display: image(imread('C.png','BackgroundColor',[1 1 1]))
  C2 : Subsystem  mask{C=51.216, IC=0, FP=Nominal, FT=0, FM=0}
      mask prompts: C "Value of C:"; IC "Initial Condition:"; FP "Fault Profile:"; FT "Fault Injection Time:"; FM "Fault Magnitude/Slope"
      mask TypeOptions FP: Nominal | Abrupt | Incipient
      mask Display: image(imread('C.png','BackgroundColor',[1 1 1]))
  C3 : Subsystem  mask{C=567.56, IC=0, FP=Nominal, FT=0, FM=0}
      mask prompts: C "Value of C:"; IC "Initial Condition:"; FP "Fault Profile:"; FT "Fault Injection Time:"; FM "Fault Magnitude/Slope"
      mask TypeOptions FP: Nominal | Abrupt | Incipient
      mask Display: image(imread('C.png','BackgroundColor',[1 1 1]))
  ComputePower : Subsystem
      mask Display: image(imread('fx.png','BackgroundColor',[1 1 1]))
  I : Subsystem
      mask Display: image(imread('1.png','BackgroundColor',[1 1 1]))
  InternalCurrent : Subsystem
      mask Display: image(imread('1.png','BackgroundColor',[1 1 1]))
  R1 : Subsystem  mask{FP=Nominal, FT=0, FM=0}
      mask prompts: FP "Fault Profile:"; FT "Fault Injection Time:"; FM "Fault Magnitude/Slope"
      mask TypeOptions FP: Nominal | Abrupt | Incipient
      mask Display: image(imread('MR.png','BackgroundColor',[1 1 1]))
  R1 Mod : Subsystem
      mask Display: image(imread('fx.png','BackgroundColor',[1 1 1]))
  R2 : Subsystem  mask{FP=Nominal, FT=0, FM=0}
      mask prompts: FP "Fault Profile:"; FT "Fault Injection Time:"; FM "Fault Magnitude/Slope"
      mask TypeOptions FP: Nominal | Abrupt | Incipient
      mask Display: image(imread('MR.png','BackgroundColor',[1 1 1]))
  R2 Mod : Subsystem
      mask Display: image(imread('fx.png','BackgroundColor',[1 1 1]))
  R3 : Subsystem  mask{FP=Nominal, FT=0, FM=0}
      mask prompts: FP "Fault Profile:"; FT "Fault Injection Time:"; FM "Fault Magnitude/Slope"
      mask TypeOptions FP: Nominal | Abrupt | Incipient
      mask Display: image(imread('MR.png','BackgroundColor',[1 1 1]))
  R3 Mod : Subsystem
      mask Display: image(imread('fx.png','BackgroundColor',[1 1 1]))
  RpDischarge : Subsystem  mask{R=500, FP=Nominal, FT=0, FM=0}
      mask prompts: R "Value of R:"; FP "Fault Profile:"; FT "Fault Injection Time:"; FM "Fault Magnitude/Slope"
      mask TypeOptions FP: Nominal | Abrupt | Incipient
      mask Display: image(imread('R.png','BackgroundColor',[1 1 1]))
  V : Subsystem
      mask Display: image(imread('0.png','BackgroundColor',[1 1 1]))
  Vp : Subsystem
      mask Display: image(imread('0.png','BackgroundColor',[1 1 1]))
  v1 : Subsystem
      mask Display: image(imread('0.png','BackgroundColor',[1 1 1]))
  v2 : Subsystem
      mask Display: image(imread('0.png','BackgroundColor',[1 1 1]))
  v3 : Subsystem
      mask Display: image(imread('0.png','BackgroundColor',[1 1 1]))
  VOutOut0 : Outport
  q : Outport  Port=2
  Voltage : Outport  Port=3
  Power : Outport  Port=4
  Current : Outport  Port=5
  wires:
  Vp/1(y(1)) -> RpDischarge/1(In)
  RpDischarge/1(Out) -> Vp/1(u(1))
  InternalCurrent/1(y(1)) -> v1/1(u(1))
  v1/1(y(1)) -> InternalCurrent/1(u(1))
  I/1(y(1)) -> V/1(u(1))
  V/1(y(1)) -> I/1(u(1))
  v1/2(y(2)) -> C1/1(F)
  C1/1(E) -> v1/2(u(2))
  Vp/2(y(2)) -> I/2(u(2))
  I/2(y(2)) -> Vp/2(u(2))
  v2/1(y(1)) -> R2/1(In)
  R2/1(Out) -> v2/1(u(1))
  v3/1(y(1)) -> R3/1(In)
  R3/1(Out) -> v3/1(u(1))
  Vp/3(y(3)) -> InternalCurrent/2(u(2))
  InternalCurrent/2(y(2)) -> Vp/3(u(3))
  InternalCurrent/3(y(3)) -> v3/2(u(2))
  v3/2(y(2)) -> InternalCurrent/3(u(3))
  Bug/1(y(1)) -> C0/1(F)
  C0/1(E) -> Bug/1(u(1))
  v3/3(y(3)) -> C3/1(F)
  C3/1(E) -> v3/3(u(3))
  v1/3(y(3)) -> R1/1(In)
  R1/1(Out) -> v1/3(u(3))
  v2/2(y(2)) -> C2/1(F)
  C2/1(E) -> v2/2(u(2))
  InternalCurrent/4(y(4)) -> Bug/2(u(2))
  Bug/2(y(2)) -> InternalCurrent/4(u(4))
  InternalCurrent/5(y(5)) -> v2/3(u(3))
  v2/3(y(3)) -> InternalCurrent/5(u(5))
  VOutIn0/1 -> V/2(u(2))
  V/2(y(2)) -> VOutOut0/1
  R3 Mod/1(y(1)) -> R3/2(RI)
  R2 Mod/1(y(1)) -> R2/2(RI)
  R1 Mod/1(y(1)) -> R1/2(RI)
  BatteryConnected/1(C) -> V/3(u(3)), I/3(u(3))
  DOC/1 -> R2 Mod/1(u(1))
  I/3(O) -> ComputePower/1(u(1)), R3 Mod/1(u(1)), Current/1
  SOC/1 -> R3 Mod/2(u(2)), R1 Mod/1(u(1))
  V/3(O) -> ComputePower/2(u(2)), Voltage/1
  ComputePower/1(y(1)) -> Power/1
  C0/3(q) -> q/1

== system VirtualADAPT/VirtualADAPTv1/PowerStorage/Battery1Subsystem/Battery1/TemperatureModel ==
  AmbientTemp : Inport
  Power : Inport  Port=2
  AmbTemp : Subsystem  mask{FP=Nominal, FT=0, FM=0}
      mask prompts: FP "Fault Profile:"; FT "Fault Injection Time:"; FM "Fault Magnitude/Slope/Bias/StuckAtValue"
      mask TypeOptions FP: Nominal | Abrupt | Incipient | Bias | StuckAt
      mask Display: image(imread('MSe.png','BackgroundColor',[1 1 1]))
  Ctheta : Subsystem  mask{C=2.2152e+006, IC=22, FP=Nominal, FT=0, FM=0}
      mask prompts: C "Value of C:"; IC "Initial Condition:"; FP "Fault Profile:"; FT "Fault Injection Time:"; FM "Fault Magnitude/Slope"
      mask TypeOptions FP: Nominal | Abrupt | Incipient
      mask Display: image(imread('C.png','BackgroundColor',[1 1 1]))
  ModAmbTemp : Subsystem
      mask Display: image(imread('fx.png','BackgroundColor',[1 1 1]))
  ModPs : Subsystem
      mask Display: image(imread('fx.png','BackgroundColor',[1 1 1]))
  OJ : Subsystem
      mask Display: image(imread('1.png','BackgroundColor',[1 1 1]))
  Rtheta : Subsystem  mask{R=0.01, FP=Nominal, FT=0, FM=0}
      mask prompts: R "Value of R:"; FP "Fault Profile:"; FT "Fault Injection Time:"; FM "Fault Magnitude/Slope"
      mask TypeOptions FP: Nominal | Abrupt | Incipient
      mask Display: image(imread('R.png','BackgroundColor',[1 1 1]))
  SfPs : Subsystem  mask{FP=Nominal, FT=0, FM=0}
      mask prompts: FP "Fault Profile:"; FT "Fault Injection Time:"; FM "Fault Magnitude/Slope/Bias/StuckAtValue"
      mask TypeOptions FP: Nominal | Abrupt | Incipient | Bias | StuckAt
      mask Display: image(imread('MSf.png','BackgroundColor',[1 1 1]))
  theta : Subsystem
      mask Display: image(imread('0.png','BackgroundColor',[1 1 1]))
  Temp : Outport
  wires:
  theta/1(y(1)) -> OJ/1(u(1))
  OJ/1(y(1)) -> theta/1(u(1))
  OJ/2(y(2)) -> Rtheta/1(In)
  Rtheta/1(Out) -> OJ/2(u(2))
  AmbTemp/1(E) -> OJ/3(u(3))
  OJ/3(y(3)) -> AmbTemp/1(F)
  theta/2(y(2)) -> Ctheta/1(F)
  SfPs/1(F) -> theta/3(u(3))
  theta/3(y(3)) -> SfPs/1(E)
  ModAmbTemp/1(y(1)) -> AmbTemp/2(Se)
  ModPs/1(y(1)) -> SfPs/2(Sf)
  Power/1 -> ModPs/1(u(1))
  AmbientTemp/1 -> ModAmbTemp/1(u(1))
  theta/4(O) -> Temp/1
  Ctheta/1(E) -> theta/2(u(2))

== system VirtualADAPT/VirtualADAPTv1/PowerStorage/Battery1Subsystem/CircuitBreakerEY136/C ==
  F : Inport
  Capacitance : Constant  Value=C
  Fault Inject : Subsystem
  Fault Profile : Constant  Value=FP
  InitialCondition : Constant  Value=IC
  Integrator : Integrator  InitialConditionSource=external
  Product1 : Product  SaturateOnIntegerOverflow=on  RndMeth=Zero  InputSameDT=on  OutDataTypeStr=Inherit: Same as first input
  Product2 : Product  SaturateOnIntegerOverflow=on  RndMeth=Zero  InputSameDT=on  OutDataTypeStr=Inherit: Same as first input
  Ramp : simulink/Sources/Ramp  slope=FM  start=FT  X0=C
  Reciprocal : Math  Operator=reciprocal  SaturateOnIntegerOverflow=on
  Step : Step  Time=FT  Before=C  After=(1 + FM)*C  SampleTime=0
  E : Outport
  C : Outport  Port=2
  q : Outport  Port=3
  wires:
  F/1 -> Integrator/1
  InitialCondition/1 -> Product1/1
  Capacitance/1 -> Fault Inject/1(Nominal), Product1/2
  Product1/1 -> Integrator/2
  Integrator/1 -> Product2/1, q/1
  Fault Inject/1(Out1) -> Reciprocal/1, C/1
  Reciprocal/1 -> Product2/2
  Fault Profile/1 -> Fault Inject/2(Fault Profile)
  Step/1 -> Fault Inject/3(Step)
  Ramp/1 -> Fault Inject/4(Ramp)
  Product2/1 -> E/1

== system VirtualADAPT/VirtualADAPTv1/PowerStorage/Battery1Subsystem/CircuitBreakerEY136/CurrentLimit ==
  Constant : Constant  Value=Constant
  Fault Inject : Subsystem
  Fault Profile : Constant  Value=FP
  Ramp : simulink/Sources/Ramp  slope=FM  start=FT  X0=Constant
  Step : Step  Time=FT  Before=Constant  After=(1 + FM)*Constant  SampleTime=0
  C : Outport
  wires:
  Fault Inject/1(Out1) -> C/1
  Constant/1 -> Fault Inject/1(Nominal)
  Fault Profile/1 -> Fault Inject/2(Fault Profile)
  Step/1 -> Fault Inject/3(Step)
  Ramp/1 -> Fault Inject/4(Ramp)
